simulink model "ospfb_2x_64kc_8i_16o"  (R2021a) (continued)
== system ospfb_2x_64kc_8i_16o/fft2/fft_direct == (continued)
      mask TypeOptions overflow: Wrap | Saturate | Error
      mask Callback use_hdl:
      | % make mutually exclusive with use_embedded.
      | 
      | use_hdl = get_param(gcb, 'use_hdl');
      | if strcmp(use_hdl, 'on'),
      |     set_param_state(gcb, 'use_embedded', 'off');
      | else
      |     set_param_state(gcb, 'use_embedded', 'on');
      | end
      mask Callback use_embedded:
      | % make mutually exclusive with use_hdl.
      | 
      | use_hdl = get_param(gcb, 'use_embedded');
      | if strcmp(use_hdl, 'on'),
      |     set_param_state(gcb, 'use_hdl', 'off');
      | else
      |     set_param_state(gcb, 'use_hdl', 'on');
      | end
      mask Callback hardcode_shifts:
      | hardcode_shifts = get_param(gcb, 'hardcode_shifts');
      | set_param_state(gcb, 'downshift', hardcode_shifts);
      mask Callback dsp48_adders: %fft_callback_dsp48_adders(gcb);
      mask DialogControl ParameterTabVar0 Prompt: Basic
      mask DialogControl ParameterTabVar01 Prompt: Latency
      mask DialogControl ParameterTabVar012 Prompt: Implementation
  butterfly3_4 : Subsystem  AncestorBlock=casper_library_ffts/butterfly_direct  mask(butterfly_direct){n_inputs=1, biplex=off, FFTSize=16, Coeffs=[ 4 16388 8196 24580 4100 20484 12292 28676 2052 18436 10244 26628 6148 22532 14340 30724 1028 17412 9220 25604 5124 21508 13316 29700 3076 19460 11268 27652 7172 23556 15364 31748 516 16900 8708 25092 4612 20996 12804 29188 2564 18948 10756 27140 6660 23044 14852 31236 1540 17924 9732 26116 5636 22020 13828 30212 3588 19972 11780 28164 7684 24068 15876 32260 260 16644 8452 24836 4356 20740 12548 28932 2308 18692 10500 26884 6404 22788 14596 30980 1284 17668 9476 25860 5380 21764 13572 29956 3332 19716 11524 27908 7428 23812 15620 32004 772 17156 8964 25348 4868 21252 13060 29444 2820 19204 11012 27396 6916 23300 15108 31492 1796 18180 9988 26372 5892 22276 14084 30468 3844 20228 12036 28420 7940 24324 16132 32516 132 16516 8324 24708 4228 20612 12420 28804 2180 18564 10372 26756 6276 22660 14468 30852 1156 17540 9348 25732 5252 21636 13444 29828 3204 19588 11396 27780 7300 23684 15492 31876 644 17028 8836 25220 4740 21124 12932 29316 2692 19076 10884 27268 6788 23172 14980 31364 1668 18052 9860 26244 5764 22148 13956 30340 3716 20100 11908 28292 7812 24196 16004 32388 388 16772 8580 24964 4484 20868 12676 29060 2436 18820 10628 27012 6532 22916 14724 31108 1412 17796 9604 25988 5508 21892 13700 30084 3460 19844 11652 28036 7556 23940 15748 32132 900 17284 9092 25476 4996 21380 13188 29572 2948 19332 11140 27524 7044 23428 15236 31620 1924 18308 10116 26500 6020 22404 14212 30596 3972 20356 12164 28548 8068 24452 16260 32644 68 16452 8260 24644 4164 20548 12356 28740 2116 18500 10308 26692 6212 22596 14404 30788 1092 17476 9284 25668 5188 21572 13380 29764 3140 19524 11332 27716 7236 23620 15428 31812 580 16964 8772 25156 4676 21060 12868 29252 2628 19012 10820 27204 6724 23108 14916 31300 1604 17988 9796 26180 5700 22084 13892 30276 3652 20036 11844 28228 7748 24132 15940 32324 324 16708 8516 24900 4420 20804 12612 28996 2372 18756 10564 26948 6468 22852 14660 31044 1348 17732 9540 25924 5444 21828 13636 30020 3396 19780 11588 27972 7492 23876 15684 32068 836 17220 9028 25412 4932 21316 13124 29508 2884 19268 11076 27460 6980 23364 15172 31556 1860 18244 10052 26436 5956 22340 14148 30532 3908 20292 12100 28484 8004 24388 16196 32580 196 16580 8388 24772 4292 20676 12484 28868 2244 18628 10436 26820 6340 22724 14532 30916 1220 17604 9412 25796 5316 21700 13508 29892 3268 19652 11460 27844 7364 23748 15556 31940 708 17092 8900 25284 4804 21188 12996 29380 2756 19140 10948 27332 6852 23236 15044 31428 1732 18116 9924 26308 5828 22212 14020 30404 3780 20164 11972 28356 7876 24260 16068 32452 452 16836 8644 25028 4548 20932 12740 29124 2500 18884 10692 27076 6596 22980 14788 31172 1476 17860 9668 26052 5572 21956 13764 30148 3524 19908 11716 28100 7620 24004 15812 32196 964 17348 9156 25540 5060 21444 13252 29636 3012 19396 11204 27588 7108 23492 15300 31684 1988 18372 10180 26564 6084 22468 14276 30660 4036 20420 12228 28612 8132 24516 16324 32708 36 16420 8228 24612 4132 20516 12324 28708 2084 18468 10276 26660 6180 22564 14372 30756 1060 17444 9252 25636 5156 21540 13348 29732 3108 19492 11300 27684 7204 23588 15396 31780 548 16932 8740 25124 4644 21028 12836 29220 2596 18980 10788 27172 6692 23076 14884 31268 1572 17956 9764 26148 5668 22052 13860 30244 3620 20004 11812 28196 7716 24100 15908 32292 292 16676 8484 24868 4388 20772 12580 28964 2340 18724 10532 26916 6436 22820 14628 31012 1316 17700 9508 25892 5412 21796 13604 29988 3364 19748 11556 27940 7460 23844 15652 32036 804 17188 8996 25380 4900 21284 13092 29476 2852 19236 11044 27428 6948 23332 15140 31524 1828 18212 10020 26404 5924 22308 14116 30500 3876 20260 12068 28452 7972 24356 16164 32548 164 16548 8356 24740 4260 20644 12452 28836 2212 18596 10404 26788 6308 22692 14500 30884 1188 17572 9380 25764 5284 21668 13476 29860 3236 19620 11428 27812 7332 23716 15524 31908 676 17060 8868 25252 4772 21156 12964 29348 2724 19108 10916 27300 6820 23204 15012 31396 1700 18084 9892 26276 5796 22180 13988 30372 3748 20132 11940 28324 7844 24228 16036 32420 420 16804 8612 ...(+19191 chars)], StepPeriod=0, coeff_bit_width=18, input_bit_width=18, bin_pt_in=17, downshift=off, async=off, floating_point=off, float_type=single, exp_width=8, frac_width=24, add_latency=1, mult_latency=3, bram_latency=2, conv_latency=1, add_pipe_latency=0, mult_pipe_latency=0, quantization=Round  (unbiased: Even Values), overflow=Wrap, coeffs_bit_limit=9, coeff_sharing=on, coeff_decimation=on, coeff_generation=off, cal_bits=1, n_bits_rotation=25, max_fanout=512, use_hdl=off, use_embedded=on, bitgrowth=off, hardcode_shifts=off, dsp48_adders=off}
      mask prompts: n_inputs "number inputs"; biplex "Used in biplex FFT"; FFTSize "Size of FFT: (2^? pts)"; Coeffs "Coefficients: (0 to 2^(FFTSize-1)-1)"; StepPeriod "Coefficient Step Period: (2^?)"; coeff_bit_width "Coefficient Bit Width"; input_bit_width "Input Bit Width"; bin_pt_in "Input binary point"; async "asynchronous operation"; floating_point "floating point"; float_type "float type"; exp_width "exponent width"; frac_width "fraction width"; add_latency "Add Latency"; mult_latency "Mult Latency"; bram_latency "BRAM Latency"; conv_latency "Convert latency"; add_pipe_latency "Add Pipeline Latency"; mult_pipe_latency "Mult Pipeline Latency"; quantization "Quantization Behavior"; overflow "Overflow Behavior"; coeffs_bit_limit "Number bits above which to store stage's coefficients in BRAM (2^? bits)"; coeff_sharing "BRAM sharing in coeff storage"; coeff_decimation "Store a fraction of coeff factors where useful"; coeff_generation "Generate coeffs with multipliers where useful"; cal_bits "Number calibration locations when generating coeffs (2^?)"; n_bits_rotation "Feedback rotation vector resolution"; max_fanout "Maximum fanout"; use_hdl "Use behavioural HDL for multipliers"; use_embedded "Use embedded multipliers"; bitgrowth "Bit growth instead of shifting"; hardcode_shifts "Hardcode shift schedule"; dsp48_adders "Use DSP48s for adders"
      mask help: FFT internal block.
      mask init: same as ospfb_2x_64kc_8i_16o/fft2/fft_direct/butterfly0_0
      settings: AttributesFormatString=twiddle_general
      mask Callback floating_point:
      | onoff = get_param(gcb, 'floating_point');
      | if strcmp(onoff,'on')
      |     enable_fixpt = 'off';
      |     set_param_state(gcb, 'float_type', 'on')
      | 
      |     f_type = get_param(gcb, 'float_type');
      |     if strcmp(f_type,'custom')
      |         set_param_state(gcb, 'exp_width', 'on')
      |         set_param_state(gcb, 'frac_width', 'on')
      |     else
      |         set_param_state(gcb, 'exp_width', 'off')
      |         set_param_state(gcb, 'frac_width', 'off')
      |     end
      | 
      | 
      | else
      |     enable_fixpt = 'on';
      |     set_param_state(gcb, 'float_type', 'off')
      |     set_param_state(gcb, 'exp_width', 'off')
      |     set_param_state(gcb, 'frac_width', 'off')
      | end
      | 
      | set_param_state(gcb, 'input_bit_width', enable_fixpt)
      | set_param_state(gcb, 'bin_pt_in', enable_fixpt)
      | set_param_state(gcb, 'coeff_bit_width', enable_fixpt)
      mask TypeOptions float_type: single | custom
      mask Callback float_type:
      | f_type = get_param(gcb, 'float_type');
      | if strcmp(f_type,'custom')
      |     set_param_state(gcb, 'exp_width', 'on')
      |     set_param_state(gcb, 'frac_width', 'on')
      | else
      |     set_param_state(gcb, 'exp_width', 'off')
      |     set_param_state(gcb, 'frac_width', 'off')
      | end
      mask TypeOptions quantization: Truncate | Round  (unbiased: +/- Inf) | Round  (unbiased: Even Values)
      mask TypeOptions overflow: Wrap | Saturate | Error
      mask Callback use_hdl:
      | % make mutually exclusive with use_embedded.
      | 
      | use_hdl = get_param(gcb, 'use_hdl');
      | if strcmp(use_hdl, 'on'),
      |     set_param_state(gcb, 'use_embedded', 'off');
      | else
      |     set_param_state(gcb, 'use_embedded', 'on');
      | end
      mask Callback use_embedded:
      | % make mutually exclusive with use_hdl.
      | 
      | use_hdl = get_param(gcb, 'use_embedded');
      | if strcmp(use_hdl, 'on'),
      |     set_param_state(gcb, 'use_hdl', 'off');
      | else
      |     set_param_state(gcb, 'use_hdl', 'on');
      | end
      mask Callback hardcode_shifts:
      | hardcode_shifts = get_param(gcb, 'hardcode_shifts');
      | set_param_state(gcb, 'downshift', hardcode_shifts);
      mask Callback dsp48_adders: %fft_callback_dsp48_adders(gcb);
      mask DialogControl ParameterTabVar0 Prompt: Basic
      mask DialogControl ParameterTabVar01 Prompt: Latency
      mask DialogControl ParameterTabVar012 Prompt: Implementation
  butterfly3_5 : Subsystem  AncestorBlock=casper_library_ffts/butterfly_direct  mask(butterfly_direct){n_inputs=1, biplex=off, FFTSize=16, Coeffs=[ 5 16389 8197 24581 4101 20485 12293 28677 2053 18437 10245 26629 6149 22533 14341 30725 1029 17413 9221 25605 5125 21509 13317 29701 3077 19461 11269 27653 7173 23557 15365 31749 517 16901 8709 25093 4613 20997 12805 29189 2565 18949 10757 27141 6661 23045 14853 31237 1541 17925 9733 26117 5637 22021 13829 30213 3589 19973 11781 28165 7685 24069 15877 32261 261 16645 8453 24837 4357 20741 12549 28933 2309 18693 10501 26885 6405 22789 14597 30981 1285 17669 9477 25861 5381 21765 13573 29957 3333 19717 11525 27909 7429 23813 15621 32005 773 17157 8965 25349 4869 21253 13061 29445 2821 19205 11013 27397 6917 23301 15109 31493 1797 18181 9989 26373 5893 22277 14085 30469 3845 20229 12037 28421 7941 24325 16133 32517 133 16517 8325 24709 4229 20613 12421 28805 2181 18565 10373 26757 6277 22661 14469 30853 1157 17541 9349 25733 5253 21637 13445 29829 3205 19589 11397 27781 7301 23685 15493 31877 645 17029 8837 25221 4741 21125 12933 29317 2693 19077 10885 27269 6789 23173 14981 31365 1669 18053 9861 26245 5765 22149 13957 30341 3717 20101 11909 28293 7813 24197 16005 32389 389 16773 8581 24965 4485 20869 12677 29061 2437 18821 10629 27013 6533 22917 14725 31109 1413 17797 9605 25989 5509 21893 13701 30085 3461 19845 11653 28037 7557 23941 15749 32133 901 17285 9093 25477 4997 21381 13189 29573 2949 19333 11141 27525 7045 23429 15237 31621 1925 18309 10117 26501 6021 22405 14213 30597 3973 20357 12165 28549 8069 24453 16261 32645 69 16453 8261 24645 4165 20549 12357 28741 2117 18501 10309 26693 6213 22597 14405 30789 1093 17477 9285 25669 5189 21573 13381 29765 3141 19525 11333 27717 7237 23621 15429 31813 581 16965 8773 25157 4677 21061 12869 29253 2629 19013 10821 27205 6725 23109 14917 31301 1605 17989 9797 26181 5701 22085 13893 30277 3653 20037 11845 28229 7749 24133 15941 32325 325 16709 8517 24901 4421 20805 12613 28997 2373 18757 10565 26949 6469 22853 14661 31045 1349 17733 9541 25925 5445 21829 13637 30021 3397 19781 11589 27973 7493 23877 15685 32069 837 17221 9029 25413 4933 21317 13125 29509 2885 19269 11077 27461 6981 23365 15173 31557 1861 18245 10053 26437 5957 22341 14149 30533 3909 20293 12101 28485 8005 24389 16197 32581 197 16581 8389 24773 4293 20677 12485 28869 2245 18629 10437 26821 6341 22725 14533 30917 1221 17605 9413 25797 5317 21701 13509 29893 3269 19653 11461 27845 7365 23749 15557 31941 709 17093 8901 25285 4805 21189 12997 29381 2757 19141 10949 27333 6853 23237 15045 31429 1733 18117 9925 26309 5829 22213 14021 30405 3781 20165 11973 28357 7877 24261 16069 32453 453 16837 8645 25029 4549 20933 12741 29125 2501 18885 10693 27077 6597 22981 14789 31173 1477 17861 9669 26053 5573 21957 13765 30149 3525 19909 11717 28101 7621 24005 15813 32197 965 17349 9157 25541 5061 21445 13253 29637 3013 19397 11205 27589 7109 23493 15301 31685 1989 18373 10181 26565 6085 22469 14277 30661 4037 20421 12229 28613 8133 24517 16325 32709 37 16421 8229 24613 4133 20517 12325 28709 2085 18469 10277 26661 6181 22565 14373 30757 1061 17445 9253 25637 5157 21541 13349 29733 3109 19493 11301 27685 7205 23589 15397 31781 549 16933 8741 25125 4645 21029 12837 29221 2597 18981 10789 27173 6693 23077 14885 31269 1573 17957 9765 26149 5669 22053 13861 30245 3621 20005 11813 28197 7717 24101 15909 32293 293 16677 8485 24869 4389 20773 12581 28965 2341 18725 10533 26917 6437 22821 14629 31013 1317 17701 9509 25893 5413 21797 13605 29989 3365 19749 11557 27941 7461 23845 15653 32037 805 17189 8997 25381 4901 21285 13093 29477 2853 19237 11045 27429 6949 23333 15141 31525 1829 18213 10021 26405 5925 22309 14117 30501 3877 20261 12069 28453 7973 24357 16165 32549 165 16549 8357 24741 4261 20645 12453 28837 2213 18597 10405 26789 6309 22693 14501 30885 1189 17573 9381 25765 5285 21669 13477 29861 3237 19621 11429 27813 7333 23717 15525 31909 677 17061 8869 25253 4773 21157 12965 29349 2725 19109 10917 27301 6821 23205 15013 31397 1701 18085 9893 26277 5797 22181 13989 30373 3749 20133 11941 28325 7845 24229 16037 32421 421 16805 8613 ...(+19191 chars)], StepPeriod=0, coeff_bit_width=18, input_bit_width=18, bin_pt_in=17, downshift=off, async=off, floating_point=off, float_type=single, exp_width=8, frac_width=24, add_latency=1, mult_latency=3, bram_latency=2, conv_latency=1, add_pipe_latency=0, mult_pipe_latency=0, quantization=Round  (unbiased: Even Values), overflow=Wrap, coeffs_bit_limit=9, coeff_sharing=on, coeff_decimation=on, coeff_generation=off, cal_bits=1, n_bits_rotation=25, max_fanout=512, use_hdl=off, use_embedded=on, bitgrowth=off, hardcode_shifts=off, dsp48_adders=off}
      mask prompts: n_inputs "number inputs"; biplex "Used in biplex FFT"; FFTSize "Size of FFT: (2^? pts)"; Coeffs "Coefficients: (0 to 2^(FFTSize-1)-1)"; StepPeriod "Coefficient Step Period: (2^?)"; coeff_bit_width "Coefficient Bit Width"; input_bit_width "Input Bit Width"; bin_pt_in "Input binary point"; async "asynchronous operation"; floating_point "floating point"; float_type "float type"; exp_width "exponent width"; frac_width "fraction width"; add_latency "Add Latency"; mult_latency "Mult Latency"; bram_latency "BRAM Latency"; conv_latency "Convert latency"; add_pipe_latency "Add Pipeline Latency"; mult_pipe_latency "Mult Pipeline Latency"; quantization "Quantization Behavior"; overflow "Overflow Behavior"; coeffs_bit_limit "Number bits above which to store stage's coefficients in BRAM (2^? bits)"; coeff_sharing "BRAM sharing in coeff storage"; coeff_decimation "Store a fraction of coeff factors where useful"; coeff_generation "Generate coeffs with multipliers where useful"; cal_bits "Number calibration locations when generating coeffs (2^?)"; n_bits_rotation "Feedback rotation vector resolution"; max_fanout "Maximum fanout"; use_hdl "Use behavioural HDL for multipliers"; use_embedded "Use embedded multipliers"; bitgrowth "Bit growth instead of shifting"; hardcode_shifts "Hardcode shift schedule"; dsp48_adders "Use DSP48s for adders"
      mask help: FFT internal block.
      mask init: same as ospfb_2x_64kc_8i_16o/fft2/fft_direct/butterfly0_0
      settings: AttributesFormatString=twiddle_general
      mask Callback floating_point:
      | onoff = get_param(gcb, 'floating_point');
      | if strcmp(onoff,'on')
      |     enable_fixpt = 'off';
      |     set_param_state(gcb, 'float_type', 'on')
      | 
      |     f_type = get_param(gcb, 'float_type');
      |     if strcmp(f_type,'custom')
      |         set_param_state(gcb, 'exp_width', 'on')
      |         set_param_state(gcb, 'frac_width', 'on')
      |     else
      |         set_param_state(gcb, 'exp_width', 'off')
      |         set_param_state(gcb, 'frac_width', 'off')
      |     end
      | 
      | 
      | else
      |     enable_fixpt = 'on';
      |     set_param_state(gcb, 'float_type', 'off')
      |     set_param_state(gcb, 'exp_width', 'off')
      |     set_param_state(gcb, 'frac_width', 'off')
      | end
      | 
      | set_param_state(gcb, 'input_bit_width', enable_fixpt)
      | set_param_state(gcb, 'bin_pt_in', enable_fixpt)
      | set_param_state(gcb, 'coeff_bit_width', enable_fixpt)
      mask TypeOptions float_type: single | custom
      mask Callback float_type:
      | f_type = get_param(gcb, 'float_type');
      | if strcmp(f_type,'custom')
      |     set_param_state(gcb, 'exp_width', 'on')
      |     set_param_state(gcb, 'frac_width', 'on')
      | else
      |     set_param_state(gcb, 'exp_width', 'off')
      |     set_param_state(gcb, 'frac_width', 'off')
      | end
      mask TypeOptions quantization: Truncate | Round  (unbiased: +/- Inf) | Round  (unbiased: Even Values)
      mask TypeOptions overflow: Wrap | Saturate | Error
      mask Callback use_hdl:
      | % make mutually exclusive with use_embedded.
      | 
      | use_hdl = get_param(gcb, 'use_hdl');
      | if strcmp(use_hdl, 'on'),
      |     set_param_state(gcb, 'use_embedded', 'off');
      | else
      |     set_param_state(gcb, 'use_embedded', 'on');
      | end
      mask Callback use_embedded:
      | % make mutually exclusive with use_hdl.
      | 
      | use_hdl = get_param(gcb, 'use_embedded');
      | if strcmp(use_hdl, 'on'),
      |     set_param_state(gcb, 'use_hdl', 'off');
      | else
      |     set_param_state(gcb, 'use_hdl', 'on');
      | end
      mask Callback hardcode_shifts:
      | hardcode_shifts = get_param(gcb, 'hardcode_shifts');
      | set_param_state(gcb, 'downshift', hardcode_shifts);
      mask Callback dsp48_adders: %fft_callback_dsp48_adders(gcb);
      mask DialogControl ParameterTabVar0 Prompt: Basic
      mask DialogControl ParameterTabVar01 Prompt: Latency
      mask DialogControl ParameterTabVar012 Prompt: Implementation
  butterfly3_6 : Subsystem  AncestorBlock=casper_library_ffts/butterfly_direct  mask(butterfly_direct){n_inputs=1, biplex=off, FFTSize=16, Coeffs=[ 6 16390 8198 24582 4102 20486 12294 28678 2054 18438 10246 26630 6150 22534 14342 30726 1030 17414 9222 25606 5126 21510 13318 29702 3078 19462 11270 27654 7174 23558 15366 31750 518 16902 8710 25094 4614 20998 12806 29190 2566 18950 10758 27142 6662 23046 14854 31238 1542 17926 9734 26118 5638 22022 13830 30214 3590 19974 11782 28166 7686 24070 15878 32262 262 16646 8454 24838 4358 20742 12550 28934 2310 18694 10502 26886 6406 22790 14598 30982 1286 17670 9478 25862 5382 21766 13574 29958 3334 19718 11526 27910 7430 23814 15622 32006 774 17158 8966 25350 4870 21254 13062 29446 2822 19206 11014 27398 6918 23302 15110 31494 1798 18182 9990 26374 5894 22278 14086 30470 3846 20230 12038 28422 7942 24326 16134 32518 134 16518 8326 24710 4230 20614 12422 28806 2182 18566 10374 26758 6278 22662 14470 30854 1158 17542 9350 25734 5254 21638 13446 29830 3206 19590 11398 27782 7302 23686 15494 31878 646 17030 8838 25222 4742 21126 12934 29318 2694 19078 10886 27270 6790 23174 14982 31366 1670 18054 9862 26246 5766 22150 13958 30342 3718 20102 11910 28294 7814 24198 16006 32390 390 16774 8582 24966 4486 20870 12678 29062 2438 18822 10630 27014 6534 22918 14726 31110 1414 17798 9606 25990 5510 21894 13702 30086 3462 19846 11654 28038 7558 23942 15750 32134 902 17286 9094 25478 4998 21382 13190 29574 2950 19334 11142 27526 7046 23430 15238 31622 1926 18310 10118 26502 6022 22406 14214 30598 3974 20358 12166 28550 8070 24454 16262 32646 70 16454 8262 24646 4166 20550 12358 28742 2118 18502 10310 26694 6214 22598 14406 30790 1094 17478 9286 25670 5190 21574 13382 29766 3142 19526 11334 27718 7238 23622 15430 31814 582 16966 8774 25158 4678 21062 12870 29254 2630 19014 10822 27206 6726 23110 14918 31302 1606 17990 9798 26182 5702 22086 13894 30278 3654 20038 11846 28230 7750 24134 15942 32326 326 16710 8518 24902 4422 20806 12614 28998 2374 18758 10566 26950 6470 22854 14662 31046 1350 17734 9542 25926 5446 21830 13638 30022 3398 19782 11590 27974 7494 23878 15686 32070 838 17222 9030 25414 4934 21318 13126 29510 2886 19270 11078 27462 6982 23366 15174 31558 1862 18246 10054 26438 5958 22342 14150 30534 3910 20294 12102 28486 8006 24390 16198 32582 198 16582 8390 24774 4294 20678 12486 28870 2246 18630 10438 26822 6342 22726 14534 30918 1222 17606 9414 25798 5318 21702 13510 29894 3270 19654 11462 27846 7366 23750 15558 31942 710 17094 8902 25286 4806 21190 12998 29382 2758 19142 10950 27334 6854 23238 15046 31430 1734 18118 9926 26310 5830 22214 14022 30406 3782 20166 11974 28358 7878 24262 16070 32454 454 16838 8646 25030 4550 20934 12742 29126 2502 18886 10694 27078 6598 22982 14790 31174 1478 17862 9670 26054 5574 21958 13766 30150 3526 19910 11718 28102 7622 24006 15814 32198 966 17350 9158 25542 5062 21446 13254 29638 3014 19398 11206 27590 7110 23494 15302 31686 1990 18374 10182 26566 6086 22470 14278 30662 4038 20422 12230 28614 8134 24518 16326 32710 38 16422 8230 24614 4134 20518 12326 28710 2086 18470 10278 26662 6182 22566 14374 30758 1062 17446 9254 25638 5158 21542 13350 29734 3110 19494 11302 27686 7206 23590 15398 31782 550 16934 8742 25126 4646 21030 12838 29222 2598 18982 10790 27174 6694 23078 14886 31270 1574 17958 9766 26150 5670 22054 13862 30246 3622 20006 11814 28198 7718 24102 15910 32294 294 16678 8486 24870 4390 20774 12582 28966 2342 18726 10534 26918 6438 22822 14630 31014 1318 17702 9510 25894 5414 21798 13606 29990 3366 19750 11558 27942 7462 23846 15654 32038 806 17190 8998 25382 4902 21286 13094 29478 2854 19238 11046 27430 6950 23334 15142 31526 1830 18214 10022 26406 5926 22310 14118 30502 3878 20262 12070 28454 7974 24358 16166 32550 166 16550 8358 24742 4262 20646 12454 28838 2214 18598 10406 26790 6310 22694 14502 30886 1190 17574 9382 25766 5286 21670 13478 29862 3238 19622 11430 27814 7334 23718 15526 31910 678 17062 8870 25254 4774 21158 12966 29350 2726 19110 10918 27302 6822 23206 15014 31398 1702 18086 9894 26278 5798 22182 13990 30374 3750 20134 11942 28326 7846 24230 16038 32422 422 16806 8614 ...(+19191 chars)], StepPeriod=0, coeff_bit_width=18, input_bit_width=18, bin_pt_in=17, downshift=off, async=off, floating_point=off, float_type=single, exp_width=8, frac_width=24, add_latency=1, mult_latency=3, bram_latency=2, conv_latency=1, add_pipe_latency=0, mult_pipe_latency=0, quantization=Round  (unbiased: Even Values), overflow=Wrap, coeffs_bit_limit=9, coeff_sharing=on, coeff_decimation=on, coeff_generation=off, cal_bits=1, n_bits_rotation=25, max_fanout=512, use_hdl=off, use_embedded=on, bitgrowth=off, hardcode_shifts=off, dsp48_adders=off}
      mask prompts: n_inputs "number inputs"; biplex "Used in biplex FFT"; FFTSize "Size of FFT: (2^? pts)"; Coeffs "Coefficients: (0 to 2^(FFTSize-1)-1)"; StepPeriod "Coefficient Step Period: (2^?)"; coeff_bit_width "Coefficient Bit Width"; input_bit_width "Input Bit Width"; bin_pt_in "Input binary point"; async "asynchronous operation"; floating_point "floating point"; float_type "float type"; exp_width "exponent width"; frac_width "fraction width"; add_latency "Add Latency"; mult_latency "Mult Latency"; bram_latency "BRAM Latency"; conv_latency "Convert latency"; add_pipe_latency "Add Pipeline Latency"; mult_pipe_latency "Mult Pipeline Latency"; quantization "Quantization Behavior"; overflow "Overflow Behavior"; coeffs_bit_limit "Number bits above which to store stage's coefficients in BRAM (2^? bits)"; coeff_sharing "BRAM sharing in coeff storage"; coeff_decimation "Store a fraction of coeff factors where useful"; coeff_generation "Generate coeffs with multipliers where useful"; cal_bits "Number calibration locations when generating coeffs (2^?)"; n_bits_rotation "Feedback rotation vector resolution"; max_fanout "Maximum fanout"; use_hdl "Use behavioural HDL for multipliers"; use_embedded "Use embedded multipliers"; bitgrowth "Bit growth instead of shifting"; hardcode_shifts "Hardcode shift schedule"; dsp48_adders "Use DSP48s for adders"
      mask help: FFT internal block.
      mask init: same as ospfb_2x_64kc_8i_16o/fft2/fft_direct/butterfly0_0
      settings: AttributesFormatString=twiddle_general
      mask Callback floating_point:
      | onoff = get_param(gcb, 'floating_point');
      | if strcmp(onoff,'on')
      |     enable_fixpt = 'off';
      |     set_param_state(gcb, 'float_type', 'on')
      | 
      |     f_type = get_param(gcb, 'float_type');
      |     if strcmp(f_type,'custom')
      |         set_param_state(gcb, 'exp_width', 'on')
      |         set_param_state(gcb, 'frac_width', 'on')
      |     else
      |         set_param_state(gcb, 'exp_width', 'off')
      |         set_param_state(gcb, 'frac_width', 'off')
      |     end
      | 
      | 
      | else
      |     enable_fixpt = 'on';
      |     set_param_state(gcb, 'float_type', 'off')
      |     set_param_state(gcb, 'exp_width', 'off')
      |     set_param_state(gcb, 'frac_width', 'off')
      | end
      | 
      | set_param_state(gcb, 'input_bit_width', enable_fixpt)
      | set_param_state(gcb, 'bin_pt_in', enable_fixpt)
      | set_param_state(gcb, 'coeff_bit_width', enable_fixpt)
      mask TypeOptions float_type: single | custom
      mask Callback float_type:
      | f_type = get_param(gcb, 'float_type');
      | if strcmp(f_type,'custom')
      |     set_param_state(gcb, 'exp_width', 'on')
      |     set_param_state(gcb, 'frac_width', 'on')
      | else
      |     set_param_state(gcb, 'exp_width', 'off')
      |     set_param_state(gcb, 'frac_width', 'off')
      | end
      mask TypeOptions quantization: Truncate | Round  (unbiased: +/- Inf) | Round  (unbiased: Even Values)
      mask TypeOptions overflow: Wrap | Saturate | Error
      mask Callback use_hdl:
      | % make mutually exclusive with use_embedded.
      | 
      | use_hdl = get_param(gcb, 'use_hdl');
      | if strcmp(use_hdl, 'on'),
      |     set_param_state(gcb, 'use_embedded', 'off');
      | else
      |     set_param_state(gcb, 'use_embedded', 'on');
      | end
      mask Callback use_embedded:
      | % make mutually exclusive with use_hdl.
      | 
      | use_hdl = get_param(gcb, 'use_embedded');
      | if strcmp(use_hdl, 'on'),
      |     set_param_state(gcb, 'use_hdl', 'off');
      | else
      |     set_param_state(gcb, 'use_hdl', 'on');
      | end
      mask Callback hardcode_shifts:
      | hardcode_shifts = get_param(gcb, 'hardcode_shifts');
      | set_param_state(gcb, 'downshift', hardcode_shifts);
      mask Callback dsp48_adders: %fft_callback_dsp48_adders(gcb);
      mask DialogControl ParameterTabVar0 Prompt: Basic
      mask DialogControl ParameterTabVar01 Prompt: Latency
      mask DialogControl ParameterTabVar012 Prompt: Implementation
  butterfly3_7 : Subsystem  AncestorBlock=casper_library_ffts/butterfly_direct  mask(butterfly_direct){n_inputs=1, biplex=off, FFTSize=16, Coeffs=[ 7 16391 8199 24583 4103 20487 12295 28679 2055 18439 10247 26631 6151 22535 14343 30727 1031 17415 9223 25607 5127 21511 13319 29703 3079 19463 11271 27655 7175 23559 15367 31751 519 16903 8711 25095 4615 20999 12807 29191 2567 18951 10759 27143 6663 23047 14855 31239 1543 17927 9735 26119 5639 22023 13831 30215 3591 19975 11783 28167 7687 24071 15879 32263 263 16647 8455 24839 4359 20743 12551 28935 2311 18695 10503 26887 6407 22791 14599 30983 1287 17671 9479 25863 5383 21767 13575 29959 3335 19719 11527 27911 7431 23815 15623 32007 775 17159 8967 25351 4871 21255 13063 29447 2823 19207 11015 27399 6919 23303 15111 31495 1799 18183 9991 26375 5895 22279 14087 30471 3847 20231 12039 28423 7943 24327 16135 32519 135 16519 8327 24711 4231 20615 12423 28807 2183 18567 10375 26759 6279 22663 14471 30855 1159 17543 9351 25735 5255 21639 13447 29831 3207 19591 11399 27783 7303 23687 15495 31879 647 17031 8839 25223 4743 21127 12935 29319 2695 19079 10887 27271 6791 23175 14983 31367 1671 18055 9863 26247 5767 22151 13959 30343 3719 20103 11911 28295 7815 24199 16007 32391 391 16775 8583 24967 4487 20871 12679 29063 2439 18823 10631 27015 6535 22919 14727 31111 1415 17799 9607 25991 5511 21895 13703 30087 3463 19847 11655 28039 7559 23943 15751 32135 903 17287 9095 25479 4999 21383 13191 29575 2951 19335 11143 27527 7047 23431 15239 31623 1927 18311 10119 26503 6023 22407 14215 30599 3975 20359 12167 28551 8071 24455 16263 32647 71 16455 8263 24647 4167 20551 12359 28743 2119 18503 10311 26695 6215 22599 14407 30791 1095 17479 9287 25671 5191 21575 13383 29767 3143 19527 11335 27719 7239 23623 15431 31815 583 16967 8775 25159 4679 21063 12871 29255 2631 19015 10823 27207 6727 23111 14919 31303 1607 17991 9799 26183 5703 22087 13895 30279 3655 20039 11847 28231 7751 24135 15943 32327 327 16711 8519 24903 4423 20807 12615 28999 2375 18759 10567 26951 6471 22855 14663 31047 1351 17735 9543 25927 5447 21831 13639 30023 3399 19783 11591 27975 7495 23879 15687 32071 839 17223 9031 25415 4935 21319 13127 29511 2887 19271 11079 27463 6983 23367 15175 31559 1863 18247 10055 26439 5959 22343 14151 30535 3911 20295 12103 28487 8007 24391 16199 32583 199 16583 8391 24775 4295 20679 12487 28871 2247 18631 10439 26823 6343 22727 14535 30919 1223 17607 9415 25799 5319 21703 13511 29895 3271 19655 11463 27847 7367 23751 15559 31943 711 17095 8903 25287 4807 21191 12999 29383 2759 19143 10951 27335 6855 23239 15047 31431 1735 18119 9927 26311 5831 22215 14023 30407 3783 20167 11975 28359 7879 24263 16071 32455 455 16839 8647 25031 4551 20935 12743 29127 2503 18887 10695 27079 6599 22983 14791 31175 1479 17863 9671 26055 5575 21959 13767 30151 3527 19911 11719 28103 7623 24007 15815 32199 967 17351 9159 25543 5063 21447 13255 29639 3015 19399 11207 27591 7111 23495 15303 31687 1991 18375 10183 26567 6087 22471 14279 30663 4039 20423 12231 28615 8135 24519 16327 32711 39 16423 8231 24615 4135 20519 12327 28711 2087 18471 10279 26663 6183 22567 14375 30759 1063 17447 9255 25639 5159 21543 13351 29735 3111 19495 11303 27687 7207 23591 15399 31783 551 16935 8743 25127 4647 21031 12839 29223 2599 18983 10791 27175 6695 23079 14887 31271 1575 17959 9767 26151 5671 22055 13863 30247 3623 20007 11815 28199 7719 24103 15911 32295 295 16679 8487 24871 4391 20775 12583 28967 2343 18727 10535 26919 6439 22823 14631 31015 1319 17703 9511 25895 5415 21799 13607 29991 3367 19751 11559 27943 7463 23847 15655 32039 807 17191 8999 25383 4903 21287 13095 29479 2855 19239 11047 27431 6951 23335 15143 31527 1831 18215 10023 26407 5927 22311 14119 30503 3879 20263 12071 28455 7975 24359 16167 32551 167 16551 8359 24743 4263 20647 12455 28839 2215 18599 10407 26791 6311 22695 14503 30887 1191 17575 9383 25767 5287 21671 13479 29863 3239 19623 11431 27815 7335 23719 15527 31911 679 17063 8871 25255 4775 21159 12967 29351 2727 19111 10919 27303 6823 23207 15015 31399 1703 18087 9895 26279 5799 22183 13991 30375 3751 20135 11943 28327 7847 24231 16039 32423 423 16807 8615 ...(+19191 chars)], StepPeriod=0, coeff_bit_width=18, input_bit_width=18, bin_pt_in=17, downshift=off, async=off, floating_point=off, float_type=single, exp_width=8, frac_width=24, add_latency=1, mult_latency=3, bram_latency=2, conv_latency=1, add_pipe_latency=0, mult_pipe_latency=0, quantization=Round  (unbiased: Even Values), overflow=Wrap, coeffs_bit_limit=9, coeff_sharing=on, coeff_decimation=on, coeff_generation=off, cal_bits=1, n_bits_rotation=25, max_fanout=512, use_hdl=off, use_embedded=on, bitgrowth=off, hardcode_shifts=off, dsp48_adders=off}
      mask prompts: n_inputs "number inputs"; biplex "Used in biplex FFT"; FFTSize "Size of FFT: (2^? pts)"; Coeffs "Coefficients: (0 to 2^(FFTSize-1)-1)"; StepPeriod "Coefficient Step Period: (2^?)"; coeff_bit_width "Coefficient Bit Width"; input_bit_width "Input Bit Width"; bin_pt_in "Input binary point"; async "asynchronous operation"; floating_point "floating point"; float_type "float type"; exp_width "exponent width"; frac_width "fraction width"; add_latency "Add Latency"; mult_latency "Mult Latency"; bram_latency "BRAM Latency"; conv_latency "Convert latency"; add_pipe_latency "Add Pipeline Latency"; mult_pipe_latency "Mult Pipeline Latency"; quantization "Quantization Behavior"; overflow "Overflow Behavior"; coeffs_bit_limit "Number bits above which to store stage's coefficients in BRAM (2^? bits)"; coeff_sharing "BRAM sharing in coeff storage"; coeff_decimation "Store a fraction of coeff factors where useful"; coeff_generation "Generate coeffs with multipliers where useful"; cal_bits "Number calibration locations when generating coeffs (2^?)"; n_bits_rotation "Feedback rotation vector resolution"; max_fanout "Maximum fanout"; use_hdl "Use behavioural HDL for multipliers"; use_embedded "Use embedded multipliers"; bitgrowth "Bit growth instead of shifting"; hardcode_shifts "Hardcode shift schedule"; dsp48_adders "Use DSP48s for adders"
      mask help: FFT internal block.
      mask init: same as ospfb_2x_64kc_8i_16o/fft2/fft_direct/butterfly0_0
      settings: AttributesFormatString=twiddle_general
      mask Callback floating_point:
      | onoff = get_param(gcb, 'floating_point');
      | if strcmp(onoff,'on')
      |     enable_fixpt = 'off';
      |     set_param_state(gcb, 'float_type', 'on')
      | 
      |     f_type = get_param(gcb, 'float_type');
      |     if strcmp(f_type,'custom')
      |         set_param_state(gcb, 'exp_width', 'on')
      |         set_param_state(gcb, 'frac_width', 'on')
      |     else
      |         set_param_state(gcb, 'exp_width', 'off')
      |         set_param_state(gcb, 'frac_width', 'off')
      |     end
      | 
      | 
      | else
      |     enable_fixpt = 'on';
      |     set_param_state(gcb, 'float_type', 'off')
      |     set_param_state(gcb, 'exp_width', 'off')
      |     set_param_state(gcb, 'frac_width', 'off')
      | end
      | 
      | set_param_state(gcb, 'input_bit_width', enable_fixpt)
      | set_param_state(gcb, 'bin_pt_in', enable_fixpt)
      | set_param_state(gcb, 'coeff_bit_width', enable_fixpt)
      mask TypeOptions float_type: single | custom
      mask Callback float_type:
      | f_type = get_param(gcb, 'float_type');
      | if strcmp(f_type,'custom')
      |     set_param_state(gcb, 'exp_width', 'on')
      |     set_param_state(gcb, 'frac_width', 'on')
      | else
      |     set_param_state(gcb, 'exp_width', 'off')
      |     set_param_state(gcb, 'frac_width', 'off')
      | end
      mask TypeOptions quantization: Truncate | Round  (unbiased: +/- Inf) | Round  (unbiased: Even Values)
      mask TypeOptions overflow: Wrap | Saturate | Error
      mask Callback use_hdl:
      | % make mutually exclusive with use_embedded.
      | 
      | use_hdl = get_param(gcb, 'use_hdl');
      | if strcmp(use_hdl, 'on'),
      |     set_param_state(gcb, 'use_embedded', 'off');
      | else
      |     set_param_state(gcb, 'use_embedded', 'on');
      | end
      mask Callback use_embedded:
      | % make mutually exclusive with use_hdl.
      | 
      | use_hdl = get_param(gcb, 'use_embedded');
      | if strcmp(use_hdl, 'on'),
      |     set_param_state(gcb, 'use_hdl', 'off');
      | else
      |     set_param_state(gcb, 'use_hdl', 'on');
      | end
      mask Callback hardcode_shifts:
      | hardcode_shifts = get_param(gcb, 'hardcode_shifts');
      | set_param_state(gcb, 'downshift', hardcode_shifts);
      mask Callback dsp48_adders: %fft_callback_dsp48_adders(gcb);
      mask DialogControl ParameterTabVar0 Prompt: Basic
      mask DialogControl ParameterTabVar01 Prompt: Latency
      mask DialogControl ParameterTabVar012 Prompt: Implementation
  combine : xbsIndex_r4/Logical  logical_function=OR  inputs=8  en=off  latency=2  precision=Full  arith_type=Unsigned  n_bits=16  bin_pt=0  align_bp=on  dbl_ovrd=off  block_type=logical
  of_0 : xbsIndex_r4/Delay  rst=off  infoeditControl=Selection of Reset will increase slice count due to use of real FFs and instead of SRLs  en=off  latency=0  reg_retiming=on  dbl_ovrd=off  block_type=delay
  of_1 : xbsIndex_r4/Concat  num_inputs=2  dbl_ovrd=off  block_type=concat
  of_2 : xbsIndex_r4/Concat  num_inputs=4  dbl_ovrd=off  block_type=concat
  of_3 : xbsIndex_r4/Concat  num_inputs=8  dbl_ovrd=off  block_type=concat
  of_expand : Subsystem  AncestorBlock=casper_library_flow_control/bus_expand  mask(bus_expand){mode=divisions of equal size, outputNum=8, outputWidth=1, outputBinaryPt=0, outputArithmeticType=0, show_format=off, outputToWorkspace=off, variablePrefix=out, outputToModelAsWell=on}
      mask prompts: outputNum "Number of outputs:"; outputWidth "Output width:"; outputBinaryPt "Output binary point position:"; outputArithmeticType "Output arithmetic type (ufix=0, fix=1, bool=2):"; show_format "Print format string?"; outputToWorkspace "Output to workspace?"; variablePrefix "Variable name prefix:"; outputToModelAsWell "Output to model as well?"
      mask help:
      | Expands a 'bus' made using a bus_create or Xilinx
      | concat block.
      | The input will be divided into the specified number
      | of outputs with the specified arithmetic types.
      | Divisions are made from most significant bit.
      mask init: same as ospfb_2x_64kc_8i_16o/bus_expand
      settings: AttributesFormatString=8 outputs
      mask TypeOptions mode: divisions of equal size | divisions of arbitrary size
      mask Callback mode: %bus_expand_callback();
      mask Callback outputWidth: %bus_expand_callback();
      mask Callback outputToWorkspace: %bus_expand_callback();
  of_or : xbsIndex_r4/Logical  logical_function=OR  inputs=4  en=off  latency=2  precision=Full  arith_type=Unsigned  n_bits=16  bin_pt=0  align_bp=on  dbl_ovrd=off  block_type=logical
  slice0 : xbsIndex_r4/Slice  nbits=1  boolean_output=on  mode=Lower Bit Location + Width  bit1=0  base1=MSB of Input  bit0=0  base0=LSB of Input  dbl_ovrd=off  block_type=slice
  slice1 : xbsIndex_r4/Slice  nbits=1  boolean_output=on  mode=Lower Bit Location + Width  bit1=0  base1=MSB of Input  bit0=1  base0=LSB of Input  dbl_ovrd=off  block_type=slice
  slice2 : xbsIndex_r4/Slice  nbits=1  boolean_output=on  mode=Lower Bit Location + Width  bit1=0  base1=MSB of Input  bit0=2  base0=LSB of Input  dbl_ovrd=off  block_type=slice
  slice3 : xbsIndex_r4/Slice  nbits=1  boolean_output=on  mode=Lower Bit Location + Width  bit1=0  base1=MSB of Input  bit0=3  base0=LSB of Input  dbl_ovrd=off  block_type=slice
  synct{1..7} (7 alike) : Terminator
  sync_out : Outport
  out00 : Outport  Port=2
  out01 : Outport  Port=3
  out02 : Outport  Port=4
  out03 : Outport  Port=5
  out04 : Outport  Port=6
  out05 : Outport  Port=7
  out06 : Outport  Port=8
  out07 : Outport  Port=9
  out08 : Outport  Port=10
  out09 : Outport  Port=11
  out010 : Outport  Port=12
  out011 : Outport  Port=13
  out012 : Outport  Port=14
  out013 : Outport  Port=15
  out014 : Outport  Port=16
  out015 : Outport  Port=17
  of : Outport  Port=18
  wires:
  in00/1 -> abus/1(in1)
  adebus3_0/1(msb_lsb_out1) -> out00/1
  in01/1 -> abus/2(in2)
  adebus3_4/1(msb_lsb_out1) -> out01/1
  in02/1 -> abus/3(in3)
  adebus3_2/1(msb_lsb_out1) -> out02/1
  in03/1 -> abus/4(in4)
  adebus3_6/1(msb_lsb_out1) -> out03/1
  in04/1 -> abus/5(in5)
  adebus3_1/1(msb_lsb_out1) -> out04/1
  in05/1 -> abus/6(in6)
  adebus3_5/1(msb_lsb_out1) -> out05/1
  in06/1 -> abus/7(in7)
  adebus3_3/1(msb_lsb_out1) -> out06/1
  in07/1 -> abus/8(in8)
  adebus3_7/1(msb_lsb_out1) -> out07/1
  in08/1 -> bbus/1(in1)
  bdebus3_0/1(msb_lsb_out1) -> out08/1
  in09/1 -> bbus/2(in2)
  bdebus3_4/1(msb_lsb_out1) -> out09/1
  in010/1 -> bbus/3(in3)
  bdebus3_2/1(msb_lsb_out1) -> out010/1
  in011/1 -> bbus/4(in4)
  bdebus3_6/1(msb_lsb_out1) -> out011/1
  in012/1 -> bbus/5(in5)
  bdebus3_1/1(msb_lsb_out1) -> out012/1
  in013/1 -> bbus/6(in6)
  bdebus3_5/1(msb_lsb_out1) -> out013/1
  in014/1 -> bbus/7(in7)
  bdebus3_3/1(msb_lsb_out1) -> out014/1
  in015/1 -> bbus/8(in8)
  bdebus3_7/1(msb_lsb_out1) -> out015/1
  of_or/1 -> of_expand/1(bus_in)
  of_expand/1(msb_out8) -> combine/1
  of_expand/2(out7) -> combine/2
  of_expand/3(out6) -> combine/3
  of_expand/4(out5) -> combine/4
  of_expand/5(out4) -> combine/5
  of_expand/6(out3) -> combine/6
  of_expand/7(out2) -> combine/7
  of_expand/8(lsb_out1) -> combine/8
  combine/1 -> of/1
  shift/1 -> slice0/1, slice1/1, slice2/1, slice3/1
  of_0/1 -> of_or/1
  sync/1 -> butterfly0_0/3(sync_in)
  slice0/1 -> butterfly0_0/4(shift)
  abus/1(bus_out) -> butterfly0_0/1(a)
  bbus/1(bus_out) -> butterfly0_0/2(b)
  butterfly0_0/3(of) -> of_0/1
  butterfly0_0/1(a+bw) -> adebus0_0/1(bus_in)
  butterfly0_0/2(a-bw) -> bdebus0_0/1(bus_in)
  of_1/1 -> of_or/2
  butterfly0_0/4(sync_out) -> butterfly1_0/3(sync_in), butterfly1_1/3(sync_in)
  slice1/1 -> butterfly1_0/4(shift), butterfly1_1/4(shift)
  adebus0_0/1(msb_out2) -> butterfly1_0/1(a)
  adebus0_0/2(lsb_out1) -> butterfly1_0/2(b)
  butterfly1_0/3(of) -> of_1/1
  butterfly1_0/1(a+bw) -> adebus1_0/1(bus_in)
  butterfly1_0/2(a-bw) -> bdebus1_0/1(bus_in)
  bdebus0_0/1(msb_out2) -> butterfly1_1/1(a)
  bdebus0_0/2(lsb_out1) -> butterfly1_1/2(b)
  butterfly1_1/3(of) -> of_1/2
  butterfly1_1/1(a+bw) -> adebus1_1/1(bus_in)
  butterfly1_1/2(a-bw) -> bdebus1_1/1(bus_in)
  of_2/1 -> of_or/3
  butterfly1_0/4(sync_out) -> butterfly2_0/3(sync_in), butterfly2_1/3(sync_in)
  slice2/1 -> butterfly2_0/4(shift), butterfly2_1/4(shift), butterfly2_2/4(shift), butterfly2_3/4(shift)
  adebus1_0/1(msb_out2) -> butterfly2_0/1(a)
  adebus1_0/2(lsb_out1) -> butterfly2_0/2(b)
  butterfly2_0/3(of) -> of_2/1
  butterfly2_0/1(a+bw) -> adebus2_0/1(bus_in)
  butterfly2_0/2(a-bw) -> bdebus2_0/1(bus_in)
  bdebus1_0/1(msb_out2) -> butterfly2_1/1(a)
  bdebus1_0/2(lsb_out1) -> butterfly2_1/2(b)
  butterfly2_1/3(of) -> of_2/2
  butterfly2_1/1(a+bw) -> adebus2_1/1(bus_in)
  butterfly2_1/2(a-bw) -> bdebus2_1/1(bus_in)
  butterfly1_1/4(sync_out) -> butterfly2_2/3(sync_in), butterfly2_3/3(sync_in)
  adebus1_1/1(msb_out2) -> butterfly2_2/1(a)
  adebus1_1/2(lsb_out1) -> butterfly2_2/2(b)
  butterfly2_2/3(of) -> of_2/3
  butterfly2_2/1(a+bw) -> adebus2_2/1(bus_in)
  butterfly2_2/2(a-bw) -> bdebus2_2/1(bus_in)
  bdebus1_1/1(msb_out2) -> butterfly2_3/1(a)
  bdebus1_1/2(lsb_out1) -> butterfly2_3/2(b)
  butterfly2_3/3(of) -> of_2/4
  butterfly2_3/1(a+bw) -> adebus2_3/1(bus_in)
  butterfly2_3/2(a-bw) -> bdebus2_3/1(bus_in)
  of_3/1 -> of_or/4
  butterfly2_0/4(sync_out) -> butterfly3_0/3(sync_in), butterfly3_1/3(sync_in)
  butterfly3_0/4(sync_out) -> sync_out/1
  slice3/1 -> butterfly3_0/4(shift), butterfly3_1/4(shift), butterfly3_2/4(shift), butterfly3_3/4(shift), butterfly3_4/4(shift), butterfly3_5/4(shift), butterfly3_6/4(shift), butterfly3_7/4(shift)
  adebus2_0/1(msb_out2) -> butterfly3_0/1(a)
  adebus2_0/2(lsb_out1) -> butterfly3_0/2(b)
  butterfly3_0/3(of) -> of_3/1
  butterfly3_1/4(sync_out) -> synct1/1
  bdebus2_0/1(msb_out2) -> butterfly3_1/1(a)
  bdebus2_0/2(lsb_out1) -> butterfly3_1/2(b)
  butterfly3_1/3(of) -> of_3/2
  butterfly2_1/4(sync_out) -> butterfly3_2/3(sync_in), butterfly3_3/3(sync_in)
  butterfly3_2/4(sync_out) -> synct2/1
  adebus2_1/1(msb_out2) -> butterfly3_2/1(a)
  adebus2_1/2(lsb_out1) -> butterfly3_2/2(b)
  butterfly3_2/3(of) -> of_3/3
  butterfly3_3/4(sync_out) -> synct3/1
  bdebus2_1/1(msb_out2) -> butterfly3_3/1(a)
  bdebus2_1/2(lsb_out1) -> butterfly3_3/2(b)
  butterfly3_3/3(of) -> of_3/4
  butterfly2_2/4(sync_out) -> butterfly3_4/3(sync_in), butterfly3_5/3(sync_in)
  butterfly3_4/4(sync_out) -> synct4/1
  adebus2_2/1(msb_out2) -> butterfly3_4/1(a)
  adebus2_2/2(lsb_out1) -> butterfly3_4/2(b)
  butterfly3_4/3(of) -> of_3/5
  butterfly3_5/4(sync_out) -> synct5/1
  bdebus2_2/1(msb_out2) -> butterfly3_5/1(a)
  bdebus2_2/2(lsb_out1) -> butterfly3_5/2(b)
  butterfly3_5/3(of) -> of_3/6
  butterfly2_3/4(sync_out) -> butterfly3_6/3(sync_in), butterfly3_7/3(sync_in)
  butterfly3_6/4(sync_out) -> synct6/1
  adebus2_3/1(msb_out2) -> butterfly3_6/1(a)
  adebus2_3/2(lsb_out1) -> butterfly3_6/2(b)
  butterfly3_6/3(of) -> of_3/7
  butterfly3_7/4(sync_out) -> synct7/1
  bdebus2_3/1(msb_out2) -> butterfly3_7/1(a)
  bdebus2_3/2(lsb_out1) -> butterfly3_7/2(b)
  butterfly3_7/3(of) -> of_3/8
  butterfly3_0/1(a+bw) -> adebus3_0/1(bus_in)
  butterfly3_0/2(a-bw) -> bdebus3_0/1(bus_in)
  butterfly3_1/1(a+bw) -> adebus3_1/1(bus_in)
  butterfly3_1/2(a-bw) -> bdebus3_1/1(bus_in)
  butterfly3_2/1(a+bw) -> adebus3_2/1(bus_in)
  butterfly3_2/2(a-bw) -> bdebus3_2/1(bus_in)
  butterfly3_3/1(a+bw) -> adebus3_3/1(bus_in)
  butterfly3_3/2(a-bw) -> bdebus3_3/1(bus_in)
  butterfly3_4/1(a+bw) -> adebus3_4/1(bus_in)
  butterfly3_4/2(a-bw) -> bdebus3_4/1(bus_in)
  butterfly3_5/1(a+bw) -> adebus3_5/1(bus_in)
  butterfly3_5/2(a-bw) -> bdebus3_5/1(bus_in)
  butterfly3_6/1(a+bw) -> adebus3_6/1(bus_in)
  butterfly3_6/2(a-bw) -> bdebus3_6/1(bus_in)
  butterfly3_7/1(a+bw) -> adebus3_7/1(bus_in)
  butterfly3_7/2(a-bw) -> bdebus3_7/1(bus_in)
